simulink model "large_model"  (Simulink 8.0)
settings: Solver=ode45  StartTime=0.0  StopTime=10.0  RelTol=1e-3
(part 12 of 21)

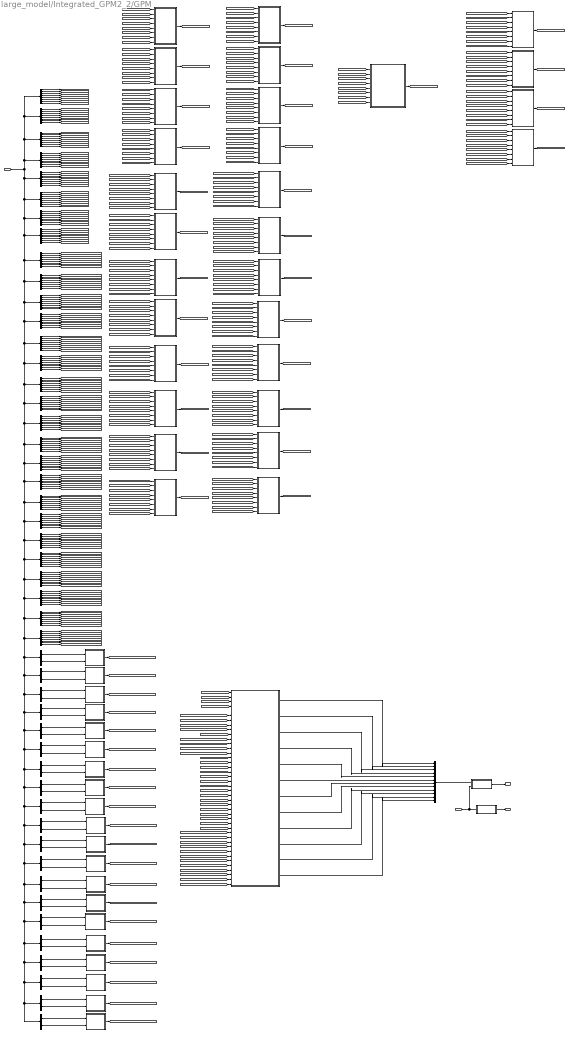
== system large_model/Integrated_GPM2_2/GPM ==
  note: Este componente foi colocado devido a uma limitação do EFTG. Na versão 1.2.1,
      | não é permitido fazer uma conexão direta entre a entrada e a saída.
  From_ES : Inport
  PWR_In : Inport  Port=2
  CAB2_FCC2 : Subsystem
  GPM Hardware : Subsystem
  Outputs : BusCreator  Inputs=12
  PWR : Subsystem
  SCP_REU_ROB_LVDT : Subsystem
  SCP_ail_REU_LIB_LVDT : Subsystem
  SCP_ail_REU_LIB_mon : Subsystem
  SCP_ail_REU_LOB_LVDT : Subsystem
  SCP_ail_REU_LOB_mon : Subsystem
  SCP_ail_REU_RIB_LVDT : Subsystem
  SCP_ail_REU_RIB_mon : Subsystem
  SCP_ail_REU_ROB_mon : Subsystem
  SCP_elev_REU_LIB_LVDT : Subsystem
  SCP_elev_REU_LIB_mon_dat : Subsystem
  SCP_elev_REU_LOB_LVDT : Subsystem
  SCP_elev_REU_LOB_mon : Subsystem
  SCP_elev_REU_RIB_LVDT_dat : Subsystem
  SCP_elev_REU_RIB_mon : Subsystem
  SCP_elev_REU_ROB_LVDT : Subsystem
  SCP_elev_REU_ROB_mon : Subsystem
  SCP_rud_REU1_LVDT : Subsystem
  SCP_rud_REU1_MON : Subsystem
  SCP_rud_REU2_LVDT : Subsystem
  SCP_rud_REU2_MON : Subsystem
  Self_checking_pair_ADS1A : Subsystem
  Self_checking_pair_ADS1B : Subsystem
  Self_checking_pair_ADS2A : Subsystem
  Self_checking_pair_ADS2B : Subsystem
  Self_checking_pair_ADS3A : Subsystem
  Self_checking_pair_ADS3B : Subsystem
  Self_checking_pair_ADS4A : Subsystem
  Self_checking_pair_ADS4B : Subsystem
  Self_checking_pair_PedalYaw1 : Subsystem
  Self_checking_pair_PedalYaw2 : Subsystem
  Self_checking_pair_PedalYaw3 : Subsystem
  Self_checking_pair_PedalYaw4 : Subsystem
  Self_checking_pair_SSC1_pitch1 : Subsystem
  Self_checking_pair_SSC1_pitch2 : Subsystem
  Self_checking_pair_SSC1_pitch3 : Subsystem
  Self_checking_pair_SSC1_pitch4 : Subsystem
  Self_checking_pair_SSC1_roll1 : Subsystem
  Self_checking_pair_SSC1_roll2 : Subsystem
  Self_checking_pair_SSC1_roll3 : Subsystem
  Self_checking_pair_SSC1_roll4 : Subsystem
  Self_checking_pair_SSC2_pitch1 : Subsystem
  Self_checking_pair_SSC2_pitch2 : Subsystem
  Self_checking_pair_SSC2_pitch3 : Subsystem
  Self_checking_pair_SSC2_pitch4 : Subsystem
  Self_checking_pair_SSC2_roll1 : Subsystem
  Self_checking_pair_SSC2_roll2 : Subsystem
  Self_checking_pair_SSC2_roll3 : Subsystem
  Self_checking_pair_SSC2_roll4 : Subsystem
  Voter_ADS : Subsystem
  ads_1A : BusSelector  Description=|  OutputSignals=switch1A.cab2_gpm_IO_chB.ads_1A,switch1A.cab1_gpm_IO_chB.ads_1A,switch1A.switch2A_to_switch.cab3_gpm_IO_chA.ads_1A,switch1A.switch2A_to_switch.cab4_gpm_IO_chA.ads_1A,switch1B.cab2_gpm_IO_chB.ads_1A,switch1B.cab1_gpm_IO_chB.ads_1A,switch1B.switch2B_to_switch.cab4_gpm_IO_chB.ads_1A,switch1B.switch2B_to_switch.cab3_gpm_IO_chB.ads_1A
      | When connected to a line which contains bus signals, this bus selector will select as its outputs all the signals which contain this bus selector name.
      | For example, if you name this bus selector as "sidestick_1_pitch_1", all signals containing this string will be selected as its outputs.
  ads_1B : BusSelector  Description=|  OutputSignals=switch1A.cab2_gpm_IO_chB.ads_1B,switch1A.cab1_gpm_IO_chB.ads_1B,switch1A.switch2A_to_switch.cab3_gpm_IO_chA.ads_1B,switch1A.switch2A_to_switch.cab4_gpm_IO_chA.ads_1B,switch1B.cab2_gpm_IO_chB.ads_1B,switch1B.cab1_gpm_IO_chB.ads_1B,switch1B.switch2B_to_switch.cab4_gpm_IO_chB.ads_1B,switch1B.switch2B_to_switch.cab3_gpm_IO_chB.ads_1B
      | When connected to a line which contains bus signals, this bus selector will select as its outputs all the signals which contain this bus selector name.
      | For example, if you name this bus selector as "sidestick_1_pitch_1", all signals containing this string will be selected as its outputs.
  ads_2A : BusSelector  Description=|  OutputSignals=switch1A.cab2_gpm_IO_chB.ads_2A,switch1A.cab1_gpm_IO_chB.ads_2A,switch1A.switch2A_to_switch.cab3_gpm_IO_chA.ads_2A,switch1A.switch2A_to_switch.cab4_gpm_IO_chA.ads_2A,switch1B.cab2_gpm_IO_chB.ads_2A,switch1B.cab1_gpm_IO_chB.ads_2A,switch1B.switch2B_to_switch.cab4_gpm_IO_chB.ads_2A,switch1B.switch2B_to_switch.cab3_gpm_IO_chB.ads_2A
      | When connected to a line which contains bus signals, this bus selector will select as its outputs all the signals which contain this bus selector name.
      | For example, if you name this bus selector as "sidestick_1_pitch_1", all signals containing this string will be selected as its outputs.
  ads_2B : BusSelector  Description=|  OutputSignals=switch1A.cab2_gpm_IO_chB.ads_2B,switch1A.cab1_gpm_IO_chB.ads_2B,switch1A.switch2A_to_switch.cab3_gpm_IO_chA.ads_2B,switch1A.switch2A_to_switch.cab4_gpm_IO_chA.ads_2B,switch1B.cab2_gpm_IO_chB.ads_2B,switch1B.cab1_gpm_IO_chB.ads_2B,switch1B.switch2B_to_switch.cab4_gpm_IO_chB.ads_2B,switch1B.switch2B_to_switch.cab3_gpm_IO_chB.ads_2B
      | When connected to a line which contains bus signals, this bus selector will select as its outputs all the signals which contain this bus selector name.
      | For example, if you name this bus selector as "sidestick_1_pitch_1", all signals containing this string will be selected as its outputs.
  ads_3A : BusSelector  Description=|  OutputSignals=switch1A.cab2_gpm_IO_chB.ads_3A,switch1A.cab1_gpm_IO_chB.ads_3A,switch1A.switch2A_to_switch.cab3_gpm_IO_chA.ads_3A,switch1A.switch2A_to_switch.cab4_gpm_IO_chA.ads_3A,switch1B.cab2_gpm_IO_chB.ads_3A,switch1B.cab1_gpm_IO_chB.ads_3A,switch1B.switch2B_to_switch.cab4_gpm_IO_chB.ads_3A,switch1B.switch2B_to_switch.cab3_gpm_IO_chB.ads_3A
      | When connected to a line which contains bus signals, this bus selector will select as its outputs all the signals which contain this bus selector name.
      | For example, if you name this bus selector as "sidestick_1_pitch_1", all signals containing this string will be selected as its outputs.
  ads_3B : BusSelector  Description=|  OutputSignals=switch1A.cab2_gpm_IO_chB.ads_3B,switch1A.cab1_gpm_IO_chB.ads_3B,switch1A.switch2A_to_switch.cab3_gpm_IO_chA.ads_3B,switch1A.switch2A_to_switch.cab4_gpm_IO_chA.ads_3B,switch1B.cab2_gpm_IO_chB.ads_3B,switch1B.cab1_gpm_IO_chB.ads_3B,switch1B.switch2B_to_switch.cab4_gpm_IO_chB.ads_3B,switch1B.switch2B_to_switch.cab3_gpm_IO_chB.ads_3B
      | When connected to a line which contains bus signals, this bus selector will select as its outputs all the signals which contain this bus selector name.
      | For example, if you name this bus selector as "sidestick_1_pitch_1", all signals containing this string will be selected as its outputs.
  ads_4A : BusSelector  Description=|  OutputSignals=switch1A.cab2_gpm_IO_chB.ads_4A,switch1A.cab1_gpm_IO_chB.ads_4A,switch1A.switch2A_to_switch.cab3_gpm_IO_chA.ads_4A,switch1A.switch2A_to_switch.cab4_gpm_IO_chA.ads_4A,switch1B.cab2_gpm_IO_chB.ads_4A,switch1B.cab1_gpm_IO_chB.ads_4A,switch1B.switch2B_to_switch.cab4_gpm_IO_chB.ads_4A,switch1B.switch2B_to_switch.cab3_gpm_IO_chB.ads_4A
      | When connected to a line which contains bus signals, this bus selector will select as its outputs all the signals which contain this bus selector name.
      | For example, if you name this bus selector as "sidestick_1_pitch_1", all signals containing this string will be selected as its outputs.
  ads_4B : BusSelector  Description=|  OutputSignals=switch1A.cab2_gpm_IO_chB.ads_4B,switch1A.cab1_gpm_IO_chB.ads_4B,switch1A.switch2A_to_switch.cab3_gpm_IO_chA.ads_4B,switch1A.switch2A_to_switch.cab4_gpm_IO_chA.ads_4B,switch1B.cab2_gpm_IO_chB.ads_4B,switch1B.cab1_gpm_IO_chB.ads_4B,switch1B.switch2B_to_switch.cab4_gpm_IO_chB.ads_4B,switch1B.switch2B_to_switch.cab3_gpm_IO_chB.ads_4B
      | When connected to a line which contains bus signals, this bus selector will select as its outputs all the signals which contain this bus selector name.
      | For example, if you name this bus selector as "sidestick_1_pitch_1", all signals containing this string will be selected as its outputs.
  ail_REU_LIB_LVDT_dat : BusSelector  OutputSignals=switch1A.switch2A_to_switch.REU_to_Switch2A.ail_REU_LIB_LVDT_dat,switch1B.switch2B_to_switch.REU_to_Switch2B.ail_REU_LIB_LVDT_dat
  ail_REU_LIB_mon_dat : BusSelector  OutputSignals=switch1A.switch2A_to_switch.REU_to_Switch2A.ail_REU_LIB_mon_dat,switch1B.switch2B_to_switch.REU_to_Switch2B.ail_REU_LIB_mon_dat
  ail_REU_LOB_LVDT_dat : BusSelector  OutputSignals=switch1A.REU_to_Switch1A.ail_REU_LOB_LVDT_dat,switch1B.REU_to_Switch1B.ail_REU_LOB_LVDT_dat
  ail_REU_LOB_mon_dat : BusSelector  OutputSignals=switch1A.REU_to_Switch1A.ail_REU_LOB_mon_dat,switch1B.REU_to_Switch1B.ail_REU_LOB_mon_dat
  ail_REU_RIB_LVDT_dat : BusSelector  OutputSignals=switch1A.switch2A_to_switch.REU_to_Switch2A.ail_REU_RIB_LVDT_dat,switch1B.switch2B_to_switch.REU_to_Switch2B.ail_REU_RIB_LVDT_dat
  ail_REU_RIB_mon_dat : BusSelector  OutputSignals=switch1A.switch2A_to_switch.REU_to_Switch2A.ail_REU_RIB_mon_dat,switch1B.switch2B_to_switch.REU_to_Switch2B.ail_REU_RIB_mon_dat
  ail_REU_ROB_LVDT_dat : BusSelector  OutputSignals=switch1A.REU_to_Switch1A.ail_REU_ROB_LVDT_dat,switch1B.REU_to_Switch1B.ail_REU_ROB_LVDT_dat
  ail_REU_ROB_mon_dat : BusSelector  OutputSignals=switch1A.REU_to_Switch1A.ail_REU_ROB_mon_dat,switch1B.REU_to_Switch1B.ail_REU_ROB_mon_dat
  elev_REU_LIB_LVDT_dat : BusSelector  OutputSignals=switch1A.switch2A_to_switch.REU_to_Switch2A.elev_REU_LIB_LVDT_dat,switch1B.switch2B_to_switch.REU_to_Switch2B.elev_REU_LIB_LVDT_dat
  elev_REU_LIB_mon_dat : BusSelector  OutputSignals=switch1A.switch2A_to_switch.REU_to_Switch2A.elev_REU_LIB_mon_dat,switch1B.switch2B_to_switch.REU_to_Switch2B.elev_REU_LIB_mon_dat
  elev_REU_LOB_LVDT_dat : BusSelector  OutputSignals=switch1A.REU_to_Switch1A.elev_REU_LOB_LVDT_dat,switch1B.REU_to_Switch1B.elev_REU_LOB_LVDT_dat
  elev_REU_LOB_mon_dat : BusSelector  OutputSignals=switch1A.REU_to_Switch1A.elev_REU_LOB_mon_dat,switch1B.REU_to_Switch1B.elev_REU_LOB_mon_dat
  elev_REU_RIB_LVDT_dat : BusSelector  OutputSignals=switch1A.switch2A_to_switch.REU_to_Switch2A.elev_REU_RIB_LVDT_dat,switch1B.switch2B_to_switch.REU_to_Switch2B.elev_REU_RIB_LVDT_dat
  elev_REU_RIB_mon_dat : BusSelector  OutputSignals=switch1A.switch2A_to_switch.REU_to_Switch2A.elev_REU_RIB_mon_dat,switch1B.switch2B_to_switch.REU_to_Switch2B.elev_REU_RIB_mon_dat
  elev_REU_ROB_LVDT_dat : BusSelector  OutputSignals=switch1A.REU_to_Switch1A.elev_REU_ROB_LVDT_dat,switch1B.REU_to_Switch1B.elev_REU_ROB_LVDT_dat
  elev_REU_ROB_mon_dat : BusSelector  OutputSignals=switch1A.REU_to_Switch1A.elev_REU_ROB_mon_dat,switch1B.REU_to_Switch1B.elev_REU_ROB_mon_dat
  pedal_yaw_1 : BusSelector  Description=|  OutputSignals=switch1A.cab2_gpm_IO_chB.rdc_4002_1.pedal_yaw_1,switch1A.cab1_gpm_IO_chB.rdc_4002_1.pedal_yaw_1,switch1A.switch2A_to_switch.cab3_gpm_IO_chA.rdc_4002_1.pedal_yaw_1,switch1A.switch2A_to_switch.cab4_gpm_IO_chA.rdc_4002_1.pedal_yaw_1,switch1B.cab2_gpm_IO_chB.rdc_4002_1.pedal_yaw_1,switch1B.cab1_gpm_IO_chB.rdc_4002_1.pedal_yaw_1,switch1B.switch2B_to_switch.cab4_gpm_IO_chB.rdc_4002_1.pedal_yaw_1,switch1B.switch2B_to_switch.cab3_gpm_IO_chB.rdc_4002_1.pedal_yaw_1
      | When connected to a line which contains bus signals, this bus selector will select as its outputs all the signals which contain this bus selector name.
      | For example, if you name this bus selector as "sidestick_1_pitch_1", all signals containing this string will be selected as its outputs.
  pedal_yaw_2 : BusSelector  Description=|  OutputSignals=switch1A.cab2_gpm_IO_chB.rdc_4002_2.pedal_yaw_2,switch1A.cab1_gpm_IO_chB.rdc_4002_2.pedal_yaw_2,switch1A.switch2A_to_switch.cab3_gpm_IO_chA.rdc_4002_2.pedal_yaw_2,switch1A.switch2A_to_switch.cab4_gpm_IO_chA.rdc_4002_2.pedal_yaw_2,switch1B.cab2_gpm_IO_chB.rdc_4002_2.pedal_yaw_2,switch1B.cab1_gpm_IO_chB.rdc_4002_2.pedal_yaw_2,switch1B.switch2B_to_switch.cab4_gpm_IO_chB.rdc_4002_2.pedal_yaw_2,switch1B.switch2B_to_switch.cab3_gpm_IO_chB.rdc_4002_2.pedal_yaw_2
      | When connected to a line which contains bus signals, this bus selector will select as its outputs all the signals which contain this bus selector name.
      | For example, if you name this bus selector as "sidestick_1_pitch_1", all signals containing this string will be selected as its outputs.
  pedal_yaw_3 : BusSelector  Description=|  OutputSignals=switch1A.cab2_gpm_IO_chB.rdc_4002_3.pedal_yaw_3,switch1A.cab1_gpm_IO_chB.rdc_4002_3.pedal_yaw_3,switch1A.switch2A_to_switch.cab3_gpm_IO_chA.rdc_4002_3.pedal_yaw_3,switch1A.switch2A_to_switch.cab4_gpm_IO_chA.rdc_4002_3.pedal_yaw_3,switch1B.cab2_gpm_IO_chB.rdc_4002_3.pedal_yaw_3,switch1B.cab1_gpm_IO_chB.rdc_4002_3.pedal_yaw_3,switch1B.switch2B_to_switch.cab4_gpm_IO_chB.rdc_4002_3.pedal_yaw_3,switch1B.switch2B_to_switch.cab3_gpm_IO_chB.rdc_4002_3.pedal_yaw_3
      | When connected to a line which contains bus signals, this bus selector will select as its outputs all the signals which contain this bus selector name.
      | For example, if you name this bus selector as "sidestick_1_pitch_1", all signals containing this string will be selected as its outputs.
  pedal_yaw_4 : BusSelector  Description=|  OutputSignals=switch1A.cab2_gpm_IO_chB.rdc_4002_4.pedal_yaw_4,switch1A.cab1_gpm_IO_chB.rdc_4002_4.pedal_yaw_4,switch1A.switch2A_to_switch.cab3_gpm_IO_chA.rdc_4002_4.pedal_yaw_4,switch1A.switch2A_to_switch.cab4_gpm_IO_chA.rdc_4002_4.pedal_yaw_4,switch1B.cab2_gpm_IO_chB.rdc_4002_4.pedal_yaw_4,switch1B.cab1_gpm_IO_chB.rdc_4002_4.pedal_yaw_4,switch1B.switch2B_to_switch.cab4_gpm_IO_chB.rdc_4002_4.pedal_yaw_4,switch1B.switch2B_to_switch.cab3_gpm_IO_chB.rdc_4002_4.pedal_yaw_4
      | When connected to a line which contains bus signals, this bus selector will select as its outputs all the signals which contain this bus selector name.
      | For example, if you name this bus selector as "sidestick_1_pitch_1", all signals containing this string will be selected as its outputs.
  rud_REU1_LVDT_dat : BusSelector  OutputSignals=switch1A.switch2A_to_switch.REU_to_Switch2A.rud_REU1_LVDT_dat,switch1B.switch2B_to_switch.REU_to_Switch2B.rud_REU1_LVDT_dat
  rud_REU1_MON_dat : BusSelector  OutputSignals=switch1A.switch2A_to_switch.REU_to_Switch2A.rud_REU1_MON_dat,switch1B.switch2B_to_switch.REU_to_Switch2B.rud_REU1_MON_dat
  rud_REU2_LVDT_dat : BusSelector  OutputSignals=switch1A.REU_to_Switch1A.rud_REU2_LVDT_dat,switch1B.REU_to_Switch1B.rud_REU2_LVDT_dat
  rud_REU2_MON_dat : BusSelector  OutputSignals=switch1A.REU_to_Switch1A.rud_REU2_MON_dat,switch1B.REU_to_Switch1B.rud_REU2_MON_dat
  sidestick1_pitch_1 : BusSelector  OutputSignals=switch1A.cab2_gpm_IO_chB.rdc_4002_1.sidesticks.sidestick1_pitch_1,switch1A.cab1_gpm_IO_chB.rdc_4002_1.sidesticks.sidestick1_pitch_1,switch1A.switch2A_to_switch.cab3_gpm_IO_chA.rdc_4002_1.sidesticks.sidestick1_pitch_1,switch1A.switch2A_to_switch.cab4_gpm_IO_chA.rdc_4002_1.sidesticks.sidestick1_pitch_1,switch1B.cab2_gpm_IO_chB.rdc_4002_1.sidesticks.sidestick1_pitch_1,switch1B.cab1_gpm_IO_chB.rdc_4002_1.sidesticks.sidestick1_pitch_1,switch1B.switch2B_to_switch.cab4_gpm_IO_chB.rdc_4002_1.sidesticks.sidestick1_pitch_1,switch1B.switch2B_to_switch.cab3_gpm_IO_chB.rdc_4002_1.sidesticks.sidestick1_pitch_1
  sidestick1_pitch_2 : BusSelector  OutputSignals=switch1A.cab2_gpm_IO_chB.rdc_4002_2.sidesticks.sidestick1_pitch_2,switch1A.cab1_gpm_IO_chB.rdc_4002_2.sidesticks.sidestick1_pitch_2,switch1A.switch2A_to_switch.cab3_gpm_IO_chA.rdc_4002_2.sidesticks.sidestick1_pitch_2,switch1A.switch2A_to_switch.cab4_gpm_IO_chA.rdc_4002_2.sidesticks.sidestick1_pitch_2,switch1B.cab2_gpm_IO_chB.rdc_4002_2.sidesticks.sidestick1_pitch_2,switch1B.cab1_gpm_IO_chB.rdc_4002_2.sidesticks.sidestick1_pitch_2,switch1B.switch2B_to_switch.cab4_gpm_IO_chB.rdc_4002_2.sidesticks.sidestick1_pitch_2,switch1B.switch2B_to_switch.cab3_gpm_IO_chB.rdc_4002_2.sidesticks.sidestick1_pitch_2
  sidestick1_pitch_3 : BusSelector  OutputSignals=switch1A.cab2_gpm_IO_chB.rdc_4002_3.sidesticks.sidestick1_pitch_3,switch1A.cab1_gpm_IO_chB.rdc_4002_3.sidesticks.sidestick1_pitch_3,switch1A.switch2A_to_switch.cab3_gpm_IO_chA.rdc_4002_3.sidesticks.sidestick1_pitch_3,switch1A.switch2A_to_switch.cab4_gpm_IO_chA.rdc_4002_3.sidesticks.sidestick1_pitch_3,switch1B.cab2_gpm_IO_chB.rdc_4002_3.sidesticks.sidestick1_pitch_3,switch1B.cab1_gpm_IO_chB.rdc_4002_3.sidesticks.sidestick1_pitch_3,switch1B.switch2B_to_switch.cab4_gpm_IO_chB.rdc_4002_3.sidesticks.sidestick1_pitch_3,switch1B.switch2B_to_switch.cab3_gpm_IO_chB.rdc_4002_3.sidesticks.sidestick1_pitch_3
  sidestick1_pitch_4 : BusSelector  OutputSignals=switch1A.cab2_gpm_IO_chB.rdc_4002_4.sidesticks.sidestick1_pitch_4,switch1A.cab1_gpm_IO_chB.rdc_4002_4.sidesticks.sidestick1_pitch_4,switch1A.switch2A_to_switch.cab3_gpm_IO_chA.rdc_4002_4.sidesticks.sidestick1_pitch_4,switch1A.switch2A_to_switch.cab4_gpm_IO_chA.rdc_4002_4.sidesticks.sidestick1_pitch_4,switch1B.cab2_gpm_IO_chB.rdc_4002_4.sidesticks.sidestick1_pitch_4,switch1B.cab1_gpm_IO_chB.rdc_4002_4.sidesticks.sidestick1_pitch_4,switch1B.switch2B_to_switch.cab4_gpm_IO_chB.rdc_4002_4.sidesticks.sidestick1_pitch_4,switch1B.switch2B_to_switch.cab3_gpm_IO_chB.rdc_4002_4.sidesticks.sidestick1_pitch_4
  sidestick1_roll_1 : BusSelector  OutputSignals=switch1A.cab2_gpm_IO_chB.rdc_4002_1.sidesticks.sidestick1_roll_1,switch1A.cab1_gpm_IO_chB.rdc_4002_1.sidesticks.sidestick1_roll_1,switch1A.switch2A_to_switch.cab3_gpm_IO_chA.rdc_4002_1.sidesticks.sidestick1_roll_1,switch1A.switch2A_to_switch.cab4_gpm_IO_chA.rdc_4002_1.sidesticks.sidestick1_roll_1,switch1B.cab2_gpm_IO_chB.rdc_4002_1.sidesticks.sidestick1_roll_1,switch1B.cab1_gpm_IO_chB.rdc_4002_1.sidesticks.sidestick1_roll_1,switch1B.switch2B_to_switch.cab4_gpm_IO_chB.rdc_4002_1.sidesticks.sidestick1_roll_1,switch1B.switch2B_to_switch.cab3_gpm_IO_chB.rdc_4002_1.sidesticks.sidestick1_roll_1
  sidestick1_roll_2 : BusSelector  OutputSignals=switch1A.cab2_gpm_IO_chB.rdc_4002_2.sidesticks.sidestick1_roll_2,switch1A.cab1_gpm_IO_chB.rdc_4002_2.sidesticks.sidestick1_roll_2,switch1A.switch2A_to_switch.cab3_gpm_IO_chA.rdc_4002_2.sidesticks.sidestick1_roll_2,switch1A.switch2A_to_switch.cab4_gpm_IO_chA.rdc_4002_2.sidesticks.sidestick1_roll_2,switch1B.cab2_gpm_IO_chB.rdc_4002_2.sidesticks.sidestick1_roll_2,switch1B.cab1_gpm_IO_chB.rdc_4002_2.sidesticks.sidestick1_roll_2,switch1B.switch2B_to_switch.cab4_gpm_IO_chB.rdc_4002_2.sidesticks.sidestick1_roll_2,switch1B.switch2B_to_switch.cab3_gpm_IO_chB.rdc_4002_2.sidesticks.sidestick1_roll_2
  sidestick1_roll_3 : BusSelector  OutputSignals=switch1A.cab2_gpm_IO_chB.rdc_4002_3.sidesticks.sidestick1_roll_3,switch1A.cab1_gpm_IO_chB.rdc_4002_3.sidesticks.sidestick1_roll_3,switch1A.switch2A_to_switch.cab3_gpm_IO_chA.rdc_4002_3.sidesticks.sidestick1_roll_3,switch1A.switch2A_to_switch.cab4_gpm_IO_chA.rdc_4002_3.sidesticks.sidestick1_roll_3,switch1B.cab2_gpm_IO_chB.rdc_4002_3.sidesticks.sidestick1_roll_3,switch1B.cab1_gpm_IO_chB.rdc_4002_3.sidesticks.sidestick1_roll_3,switch1B.switch2B_to_switch.cab4_gpm_IO_chB.rdc_4002_3.sidesticks.sidestick1_roll_3,switch1B.switch2B_to_switch.cab3_gpm_IO_chB.rdc_4002_3.sidesticks.sidestick1_roll_3
  sidestick1_roll_4 : BusSelector  OutputSignals=switch1A.cab2_gpm_IO_chB.rdc_4002_4.sidesticks.sidestick1_roll_4,switch1A.cab1_gpm_IO_chB.rdc_4002_4.sidesticks.sidestick1_roll_4,switch1A.switch2A_to_switch.cab3_gpm_IO_chA.rdc_4002_4.sidesticks.sidestick1_roll_4,switch1A.switch2A_to_switch.cab4_gpm_IO_chA.rdc_4002_4.sidesticks.sidestick1_roll_4,switch1B.cab2_gpm_IO_chB.rdc_4002_4.sidesticks.sidestick1_roll_4,switch1B.cab1_gpm_IO_chB.rdc_4002_4.sidesticks.sidestick1_roll_4,switch1B.switch2B_to_switch.cab4_gpm_IO_chB.rdc_4002_4.sidesticks.sidestick1_roll_4,switch1B.switch2B_to_switch.cab3_gpm_IO_chB.rdc_4002_4.sidesticks.sidestick1_roll_4
  sidestick2_pitch_1 : BusSelector  OutputSignals=switch1A.cab2_gpm_IO_chB.rdc_4002_1.sidesticks.sidestick2_pitch_1,switch1A.cab1_gpm_IO_chB.rdc_4002_1.sidesticks.sidestick2_pitch_1,switch1A.switch2A_to_switch.cab3_gpm_IO_chA.rdc_4002_1.sidesticks.sidestick2_pitch_1,switch1A.switch2A_to_switch.cab4_gpm_IO_chA.rdc_4002_1.sidesticks.sidestick2_pitch_1,switch1B.cab2_gpm_IO_chB.rdc_4002_1.sidesticks.sidestick2_pitch_1,switch1B.cab1_gpm_IO_chB.rdc_4002_1.sidesticks.sidestick2_pitch_1,switch1B.switch2B_to_switch.cab4_gpm_IO_chB.rdc_4002_1.sidesticks.sidestick2_pitch_1,switch1B.switch2B_to_switch.cab3_gpm_IO_chB.rdc_4002_1.sidesticks.sidestick2_pitch_1
  sidestick2_pitch_2 : BusSelector  OutputSignals=switch1A.cab2_gpm_IO_chB.rdc_4002_2.sidesticks.sidestick2_pitch_2,switch1A.cab1_gpm_IO_chB.rdc_4002_2.sidesticks.sidestick2_pitch_2,switch1A.switch2A_to_switch.cab3_gpm_IO_chA.rdc_4002_2.sidesticks.sidestick2_pitch_2,switch1A.switch2A_to_switch.cab4_gpm_IO_chA.rdc_4002_2.sidesticks.sidestick2_pitch_2,switch1B.cab2_gpm_IO_chB.rdc_4002_2.sidesticks.sidestick2_pitch_2,switch1B.cab1_gpm_IO_chB.rdc_4002_2.sidesticks.sidestick2_pitch_2,switch1B.switch2B_to_switch.cab4_gpm_IO_chB.rdc_4002_2.sidesticks.sidestick2_pitch_2,switch1B.switch2B_to_switch.cab3_gpm_IO_chB.rdc_4002_2.sidesticks.sidestick2_pitch_2
  sidestick2_pitch_3 : BusSelector  OutputSignals=switch1A.cab2_gpm_IO_chB.rdc_4002_3.sidesticks.sidestick2_pitch_3,switch1A.cab1_gpm_IO_chB.rdc_4002_3.sidesticks.sidestick2_pitch_3,switch1A.switch2A_to_switch.cab3_gpm_IO_chA.rdc_4002_3.sidesticks.sidestick2_pitch_3,switch1A.switch2A_to_switch.cab4_gpm_IO_chA.rdc_4002_3.sidesticks.sidestick2_pitch_3,switch1B.cab2_gpm_IO_chB.rdc_4002_3.sidesticks.sidestick2_pitch_3,switch1B.cab1_gpm_IO_chB.rdc_4002_3.sidesticks.sidestick2_pitch_3,switch1B.switch2B_to_switch.cab4_gpm_IO_chB.rdc_4002_3.sidesticks.sidestick2_pitch_3,switch1B.switch2B_to_switch.cab3_gpm_IO_chB.rdc_4002_3.sidesticks.sidestick2_pitch_3
  sidestick2_pitch_4 : BusSelector  OutputSignals=switch1A.cab2_gpm_IO_chB.rdc_4002_4.sidesticks.sidestick2_pitch_4,switch1A.cab1_gpm_IO_chB.rdc_4002_4.sidesticks.sidestick2_pitch_4,switch1A.switch2A_to_switch.cab3_gpm_IO_chA.rdc_4002_4.sidesticks.sidestick2_pitch_4,switch1A.switch2A_to_switch.cab4_gpm_IO_chA.rdc_4002_4.sidesticks.sidestick2_pitch_4,switch1B.cab2_gpm_IO_chB.rdc_4002_4.sidesticks.sidestick2_pitch_4,switch1B.cab1_gpm_IO_chB.rdc_4002_4.sidesticks.sidestick2_pitch_4,switch1B.switch2B_to_switch.cab4_gpm_IO_chB.rdc_4002_4.sidesticks.sidestick2_pitch_4,switch1B.switch2B_to_switch.cab3_gpm_IO_chB.rdc_4002_4.sidesticks.sidestick2_pitch_4
  sidestick2_roll_1 : BusSelector  OutputSignals=switch1A.cab2_gpm_IO_chB.rdc_4002_1.sidesticks.sidestick2_roll_1,switch1A.cab1_gpm_IO_chB.rdc_4002_1.sidesticks.sidestick2_roll_1,switch1A.switch2A_to_switch.cab3_gpm_IO_chA.rdc_4002_1.sidesticks.sidestick2_roll_1,switch1A.switch2A_to_switch.cab4_gpm_IO_chA.rdc_4002_1.sidesticks.sidestick2_roll_1,switch1B.cab2_gpm_IO_chB.rdc_4002_1.sidesticks.sidestick2_roll_1,switch1B.cab1_gpm_IO_chB.rdc_4002_1.sidesticks.sidestick2_roll_1,switch1B.switch2B_to_switch.cab4_gpm_IO_chB.rdc_4002_1.sidesticks.sidestick2_roll_1,switch1B.switch2B_to_switch.cab3_gpm_IO_chB.rdc_4002_1.sidesticks.sidestick2_roll_1
  sidestick2_roll_2 : BusSelector  OutputSignals=switch1A.cab2_gpm_IO_chB.rdc_4002_2.sidesticks.sidestick2_roll_2,switch1A.cab1_gpm_IO_chB.rdc_4002_2.sidesticks.sidestick2_roll_2,switch1A.switch2A_to_switch.cab3_gpm_IO_chA.rdc_4002_2.sidesticks.sidestick2_roll_2,switch1A.switch2A_to_switch.cab4_gpm_IO_chA.rdc_4002_2.sidesticks.sidestick2_roll_2,switch1B.cab2_gpm_IO_chB.rdc_4002_2.sidesticks.sidestick2_roll_2,switch1B.cab1_gpm_IO_chB.rdc_4002_2.sidesticks.sidestick2_roll_2,switch1B.switch2B_to_switch.cab4_gpm_IO_chB.rdc_4002_2.sidesticks.sidestick2_roll_2,switch1B.switch2B_to_switch.cab3_gpm_IO_chB.rdc_4002_2.sidesticks.sidestick2_roll_2
  sidestick2_roll_3 : BusSelector  OutputSignals=switch1A.cab2_gpm_IO_chB.rdc_4002_3.sidesticks.sidestick2_roll_3,switch1A.cab1_gpm_IO_chB.rdc_4002_3.sidesticks.sidestick2_roll_3,switch1A.switch2A_to_switch.cab3_gpm_IO_chA.rdc_4002_3.sidesticks.sidestick2_roll_3,switch1A.switch2A_to_switch.cab4_gpm_IO_chA.rdc_4002_3.sidesticks.sidestick2_roll_3,switch1B.cab2_gpm_IO_chB.rdc_4002_3.sidesticks.sidestick2_roll_3,switch1B.cab1_gpm_IO_chB.rdc_4002_3.sidesticks.sidestick2_roll_3,switch1B.switch2B_to_switch.cab4_gpm_IO_chB.rdc_4002_3.sidesticks.sidestick2_roll_3,switch1B.switch2B_to_switch.cab3_gpm_IO_chB.rdc_4002_3.sidesticks.sidestick2_roll_3
  sidestick2_roll_4 : BusSelector  OutputSignals=switch1A.cab2_gpm_IO_chB.rdc_4002_4.sidesticks.sidestick2_roll_4,switch1A.cab1_gpm_IO_chB.rdc_4002_4.sidesticks.sidestick2_roll_4,switch1A.switch2A_to_switch.cab3_gpm_IO_chA.rdc_4002_4.sidesticks.sidestick2_roll_4,switch1A.switch2A_to_switch.cab4_gpm_IO_chA.rdc_4002_4.sidesticks.sidestick2_roll_4,switch1B.cab2_gpm_IO_chB.rdc_4002_4.sidesticks.sidestick2_roll_4,switch1B.cab1_gpm_IO_chB.rdc_4002_4.sidesticks.sidestick2_roll_4,switch1B.switch2B_to_switch.cab4_gpm_IO_chB.rdc_4002_4.sidesticks.sidestick2_roll_4,switch1B.switch2B_to_switch.cab3_gpm_IO_chB.rdc_4002_4.sidesticks.sidestick2_roll_4
  To_ES : Outport
  PWR_Out : Outport  Port=2
  wires:
  CAB2_FCC2/12(fcc_ram_mon_ELEV_ROB_dat) -> Outputs/12  "CAB2_GPM2_fcc_ram_mon_ELEV_ROB_dat"
  CAB2_FCC2/11(fcc_ram_mon_ELEV_RIB_dat) -> Outputs/11  "CAB2_GPM2_fcc_ram_mon_ELEV_RIB_dat"
  CAB2_FCC2/10(fcc_ram_mon_ELEV_LOB_dat) -> Outputs/10  "CAB2_GPM2_fcc_ram_mon_ELEV_LOB_dat"
  CAB2_FCC2/9(fcc_ram_mon_ELEV_LIB_dat) -> Outputs/9  "CAB2_GPM2_fcc_ram_mon_ELEV_LIB_dat"
  CAB2_FCC2/8(fcc_ram_mon_AIL_ROB_dat) -> Outputs/8  "CAB2_GPM2_fcc_ram_mon_AIL_ROB_dat"
  CAB2_FCC2/7(fcc_ram_mon_AIL_RIB_dat) -> Outputs/7  "CAB2_GPM2_fcc_ram_mon_AIL_RIB_dat"
  CAB2_FCC2/6(fcc_ram_mon_AIL_LOB_dat) -> Outputs/6  "CAB2_GPM2_fcc_ram_mon_AIL_LOB_dat"
  CAB2_FCC2/5(fcc_ram_mon_AIL_LIB_dat) -> Outputs/5  "CAB2_GPM2_fcc_ram_mon_AIL_LIB_dat"
  CAB2_FCC2/4(fcc_ram_mon_rudder_UPR_dat) -> Outputs/4  "CAB2_GPM2_fcc_ram_mon_rudder_UPR_dat"
  CAB2_FCC2/3(fcc_ram_mon_rudder_LWR_dat) -> Outputs/3  "CAB2_GPM2_fcc_ram_mon_rudder_LWR_dat"
  CAB2_FCC2/2(pitch_roll_cmd) -> Outputs/2  "CAB2_GPM2_pitch_roll_cmd"
  CAB2_FCC2/1(yaw_cmd) -> Outputs/1  "CAB2_GPM2_yaw_cmd"
  [SCP_elev_REU_RIB_LVDT_dat_1] -> CAB2_FCC2/39(REU_ELEV_LVDT_RIB_dat)
  SCP_elev_REU_RIB_LVDT_dat/1(Out) -> [SCP_elev_REU_RIB_LVDT_dat_1]
  [SCP_elev_REU_RIB_mon_1] -> CAB2_FCC2/40(REU_ELEV_mon_RIB_dat)
  SCP_elev_REU_RIB_mon/1(Out) -> [SCP_elev_REU_RIB_mon_1]
  [SCP_elev_REU_ROB_LVDT_1] -> CAB2_FCC2/41(REU_ELEV_LVDT_ROB_dat)
  SCP_elev_REU_ROB_LVDT/1(Out) -> [SCP_elev_REU_ROB_LVDT_1]
  [SCP_elev_REU_ROB_mon_1] -> CAB2_FCC2/42(REU_ELEV_mon_ROB_dat)
  SCP_elev_REU_ROB_mon/1(Out) -> [SCP_elev_REU_ROB_mon_1]
  [SCP_elev_REU_LOB_LVDT_1] -> CAB2_FCC2/33(REU_ELEV_LVDT_LOB_dat)
  SCP_elev_REU_LOB_LVDT/1(Out) -> [SCP_elev_REU_LOB_LVDT_1]
  [SCP_elev_REU_LOB_mon_1] -> CAB2_FCC2/34(REU_ELEV_mon_LOB_dat)
  SCP_elev_REU_LOB_mon/1(Out) -> [SCP_elev_REU_LOB_mon_1]
  [SCP_rud_REU1_LVDT_1] -> CAB2_FCC2/6(REU1_LVDT_rudder_dat)
  SCP_rud_REU1_LVDT/1(Out) -> [SCP_rud_REU1_LVDT_1]
  [SCP_rud_REU1_MON_1] -> CAB2_FCC2/7(REU1_mon_rudder_dat)
  SCP_rud_REU1_MON/1(Out) -> [SCP_rud_REU1_MON_1]
  [SCP_rud_REU2_LVDT_1] -> CAB2_FCC2/8(REU2_LVDT_rudder_dat)
  SCP_rud_REU2_LVDT/1(Out) -> [SCP_rud_REU2_LVDT_1]
  [SCP_rud_REU2_MON_1] -> CAB2_FCC2/9(REU2_mon_rudder_dat)
  SCP_rud_REU2_MON/1(Out) -> [SCP_rud_REU2_MON_1]
  [SCP_ail_REU_LIB_mon_1] -> CAB2_FCC2/12(REU_mon_AIL_LIB_dat)
  SCP_ail_REU_LIB_mon/1(Out) -> [SCP_ail_REU_LIB_mon_1]
  [SCP_ail_REU_LOB_LVDT_1] -> CAB2_FCC2/13(REU_LVDT_AIL_LOB_dat)
  SCP_ail_REU_LOB_LVDT/1(Out) -> [SCP_ail_REU_LOB_LVDT_1]
  [SCP_ail_REU_LOB_mon_1] -> CAB2_FCC2/14(REU_mon_AIL_LOB_dat)
  SCP_ail_REU_LOB_mon/1(Out) -> [SCP_ail_REU_LOB_mon_1]
  [SCP_REU_ROB_LVDT_1] -> CAB2_FCC2/37(REU_LVDT_AIL_ROB_dat)
  SCP_REU_ROB_LVDT/1(Out) -> [SCP_REU_ROB_LVDT_1]
  [SCP_ail_REU_ROB_mon_1] -> CAB2_FCC2/38(REU_mon_AIL_ROB_dat)
  SCP_ail_REU_ROB_mon/1(Out) -> [SCP_ail_REU_ROB_mon_1]
  [SCP_elev_REU_LIB_LVDT_1] -> CAB2_FCC2/31(REU_ELEV_LVDT_LIB_dat)
  SCP_elev_REU_LIB_LVDT/1(Out) -> [SCP_elev_REU_LIB_LVDT_1]
  [SCP_elev_REU_LIB_mon_dat_1] -> CAB2_FCC2/32(REU_ELEV_mon_LIB_dat)
  SCP_elev_REU_LIB_mon_dat/1(Out) -> [SCP_elev_REU_LIB_mon_dat_1]
  [SCP_ail_REU_RIB_LVDT_1] -> CAB2_FCC2/35(REU_LVDT_AIL_RIB_dat)
  SCP_ail_REU_RIB_LVDT/1(Out) -> [SCP_ail_REU_RIB_LVDT_1]
  [SCP_ail_REU_RIB_mon_1] -> CAB2_FCC2/36(REU_mon_AIL_RIB_dat)
  SCP_ail_REU_RIB_mon/1(Out) -> [SCP_ail_REU_RIB_mon_1]
  [SCP_ail_REU_LIB_LVDT_1] -> CAB2_FCC2/11(REU_LVDT_AIL_LIB_dat)
  SCP_ail_REU_LIB_LVDT/1(Out) -> [SCP_ail_REU_LIB_LVDT_1]
  elev_REU_ROB_mon_dat/2 -> SCP_elev_REU_ROB_mon/2(In2)  "<elev_REU_ROB_mon_dat>"
  elev_REU_ROB_mon_dat/1 -> SCP_elev_REU_ROB_mon/1(In1)  "<elev_REU_ROB_mon_dat>"
  elev_REU_ROB_LVDT_dat/2 -> SCP_elev_REU_ROB_LVDT/2(In2)  "<elev_REU_ROB_LVDT_dat>"
  elev_REU_ROB_LVDT_dat/1 -> SCP_elev_REU_ROB_LVDT/1(In1)  "<elev_REU_ROB_LVDT_dat>"
  elev_REU_RIB_mon_dat/2 -> SCP_elev_REU_RIB_mon/2(In2)  "<elev_REU_RIB_mon_dat>"
  elev_REU_RIB_mon_dat/1 -> SCP_elev_REU_RIB_mon/1(In1)  "<elev_REU_RIB_mon_dat>"
  elev_REU_RIB_LVDT_dat/2 -> SCP_elev_REU_RIB_LVDT_dat/2(In2)  "<elev_REU_RIB_LVDT_dat>"
  elev_REU_RIB_LVDT_dat/1 -> SCP_elev_REU_RIB_LVDT_dat/1(In1)  "<elev_REU_RIB_LVDT_dat>"
  [ads_dat] -> CAB2_FCC2/10(ads_dat)
  [PedalYaw1_cmd] -> CAB2_FCC2/1(PedalYaw1_cmd)
  [PedalYaw2_cmd] -> CAB2_FCC2/2(PedalYaw2_cmd)
  [PedalYaw3_cmd] -> CAB2_FCC2/3(PedalYaw3_cmd)
  [PedalYaw4_cmd] -> CAB2_FCC2/4(PedalYaw4_cmd)
  [sidestick1Pitch1_cmd] -> CAB2_FCC2/15(sidestick1Pitch1_cmd)
  [sidestick1Pitch2_cmd] -> CAB2_FCC2/16(sidestick1Pitch2_cmd)
  [sidestick1Pitch3_cmd] -> CAB2_FCC2/17(sidestick1Pitch3_cmd)
  [sidestick1Pitch4_cmd] -> CAB2_FCC2/18(sidestick1Pitch4_cmd)
  [sidestick1Roll1_cmd] -> CAB2_FCC2/19(sidestick1Roll1_cmd)
  [sidestick1Roll2_cmd] -> CAB2_FCC2/20(sidestick1Roll2_cmd)
  [sidestick1Roll3_cmd] -> CAB2_FCC2/21(sidestick1Roll3_cmd)
  [sidestick1Roll4_cmd] -> CAB2_FCC2/22(sidestick1Roll4_cmd)
  [sidestick2Pitch1_cmd] -> CAB2_FCC2/23(sidestick2Pitch1_cmd)
  [sidestick2Pitch2_cmd] -> CAB2_FCC2/24(sidestick2Pitch2_cmd)
  [sidestick2Pitch3_cmd] -> CAB2_FCC2/25(sidestick2Pitch3_cmd)
  [sidestick2Pitch4_cmd] -> CAB2_FCC2/26(sidestick2Pitch4_cmd)
  [sidestick2Roll1_cmd] -> CAB2_FCC2/27(sidestick2Roll1_cmd)
  [sidestick2Roll2_cmd] -> CAB2_FCC2/28(sidestick2Roll2_cmd)
  [sidestick2Roll3_cmd] -> CAB2_FCC2/29(sidestick2Roll3_cmd)
  [sidestick2Roll4_cmd] -> CAB2_FCC2/30(sidestick2Roll4_cmd)
  PWR/1(Out1) -> PWR_Out/1
  GPM Hardware/1(Out) -> To_ES/1
  PWR_In/1 -> PWR/1(In1), GPM Hardware/2(PWR_In)
  Outputs/1 -> GPM Hardware/1(Data)
  Voter_ADS/1(ADS) -> [ads_dat]
  Self_checking_pair_ADS1A/1(Out) -> [Self_checking_pair_ADS1A_1_]
  [Self_checking_pair_ADS1A_1_] -> Voter_ADS/1(ADS1A)
  Self_checking_pair_ADS2B/1(Out) -> [Self_checking_pair_ADS2B_1_]
  [Self_checking_pair_ADS2B_1_] -> Voter_ADS/4(ADS2B)
  Self_checking_pair_ADS2A/1(Out) -> [Self_checking_pair_ADS2A_1_]
  [Self_checking_pair_ADS2A_1_] -> Voter_ADS/3(ADS2A)
  Self_checking_pair_ADS1B/1(Out) -> [Self_checking_pair_ADS1B_1_]
  [Self_checking_pair_ADS1B_1_] -> Voter_ADS/2(ADS1B)
  Self_checking_pair_ADS4B/1(Out) -> [Self_checking_pair_ADS4B_1_]
  [Self_checking_pair_ADS4B_1_] -> Voter_ADS/8(ADS4B)
  [Self_checking_pair_ADS4A_1_] -> Voter_ADS/7(ADS4A)
  Self_checking_pair_ADS3B/1(Out) -> [Self_checking_pair_ADS3B_1_]
  [Self_checking_pair_ADS3B_1_] -> Voter_ADS/6(ADS3B)
  [Self_checking_pair_ADS3A_1_] -> Voter_ADS/5(ADS3A)
  Self_checking_pair_ADS3A/1(Out) -> [Self_checking_pair_ADS3A_1_]
  Self_checking_pair_ADS4A/1(Out) -> [Self_checking_pair_ADS4A_1_]
  Self_checking_pair_PedalYaw1/1(Out) -> [PedalYaw1_cmd]
  Self_checking_pair_PedalYaw2/1(Out) -> [PedalYaw2_cmd]
  Self_checking_pair_PedalYaw3/1(Out) -> [PedalYaw3_cmd]
  Self_checking_pair_PedalYaw4/1(Out) -> [PedalYaw4_cmd]
  From_ES/1 -> ads_1A/1, ads_1B/1, ads_2A/1, ads_2B/1, sidestick1_pitch_2/1, sidestick1_pitch_3/1, sidestick2_pitch_1/1, sidestick2_pitch_2/1, sidestick2_pitch_3/1, sidestick2_pitch_4/1, sidestick1_roll_1/1, sidestick1_roll_2/1, sidestick1_roll_3/1, sidestick1_roll_4/1, sidestick2_roll_1/1, sidestick2_roll_2/1, sidestick2_roll_3/1, sidestick2_roll_4/1, ail_REU_LIB_LVDT_dat/1, ail_REU_LIB_mon_dat/1, ail_REU_LOB_LVDT_dat/1, ail_REU_LOB_mon_dat/1, ail_REU_RIB_LVDT_dat/1, ail_REU_RIB_mon_dat/1, ail_REU_ROB_LVDT_dat/1, ail_REU_ROB_mon_dat/1, elev_REU_LIB_LVDT_dat/1, elev_REU_LIB_mon_dat/1, elev_REU_LOB_LVDT_dat/1, elev_REU_LOB_mon_dat/1, rud_REU1_LVDT_dat/1, rud_REU1_MON_dat/1, elev_REU_ROB_mon_dat/1, elev_REU_ROB_LVDT_dat/1, elev_REU_RIB_mon_dat/1, elev_REU_RIB_LVDT_dat/1, rud_REU2_MON_dat/1, rud_REU2_LVDT_dat/1, sidestick1_pitch_4/1, sidestick1_pitch_1/1, pedal_yaw_4/1, pedal_yaw_3/1, pedal_yaw_2/1, pedal_yaw_1/1, ads_4B/1, ads_4A/1, ads_3B/1, ads_3A/1
  ads_4B/8 -> [ADS4B_8_ads_4B]  "<ads_4B>"
  [ADS4B_8_ads_4B] -> Self_checking_pair_ADS4B/8(In8)
  ads_4B/7 -> [ADS4B_7_ads_4B]  "<ads_4B>"
  [ADS4B_7_ads_4B] -> Self_checking_pair_ADS4B/7(In7)
  ads_4B/6 -> [ADS4B_6_ads_4B]  "<ads_4B>"
  [ADS4B_6_ads_4B] -> Self_checking_pair_ADS4B/6(In6)
  ads_4B/5 -> [ADS4B_5_ads_4B]  "<ads_4B>"
  [ADS4B_5_ads_4B] -> Self_checking_pair_ADS4B/5(In5)
  ads_4B/4 -> [ADS4B_4_ads_4B]  "<ads_4B>"
  [ADS4B_4_ads_4B] -> Self_checking_pair_ADS4B/4(In4)
  ads_4B/3 -> [ADS4B_3_ads_4B]  "<ads_4B>"
  [ADS4B_3_ads_4B] -> Self_checking_pair_ADS4B/3(In3)
  ads_4B/2 -> [ADS4B_2_ads_4B]  "<ads_4B>"
  [ADS4B_2_ads_4B] -> Self_checking_pair_ADS4B/2(In2)
  ads_4B/1 -> [ADS4B_1_ads_4B]  "<ads_4B>"
  [ADS4B_1_ads_4B] -> Self_checking_pair_ADS4B/1(In1)
  ads_4A/8 -> [ADS4A_8_ads_4A]  "<ads_4A>"
  [ADS4A_8_ads_4A] -> Self_checking_pair_ADS4A/8(In8)
  ads_4A/7 -> [ADS4A_7_ads_4A]  "<ads_4A>"
  [ADS4A_7_ads_4A] -> Self_checking_pair_ADS4A/7(In7)
  ads_4A/6 -> [ADS4A_6_ads_4A]  "<ads_4A>"
  [ADS4A_6_ads_4A] -> Self_checking_pair_ADS4A/6(In6)
  ads_4A/5 -> [ADS4A_5_ads_4A]  "<ads_4A>"
  [ADS4A_5_ads_4A] -> Self_checking_pair_ADS4A/5(In5)
  ads_4A/4 -> [ADS4A_4_ads_4A]  "<ads_4A>"
  [ADS4A_4_ads_4A] -> Self_checking_pair_ADS4A/4(In4)